simulink model "BaseEngineController_A02_bak2"  (Simulink 7.7)
settings: Solver=FixedStepDiscrete  StartTime=0.0  StopTime=10.0  RelTol=1e-3
PreLoadFcn:
  | try, eval([bdroot '_setup']); catch, eval('model_setup', ''); end
InitFcn:
  | try, eval([bdroot '_setup']); catch, eval('model_setup', ''); end
undefined symbols (not defined in the file or in its folder): ECU555, High, motohawk_build_version, motohawk_trunc_name, motohawk_vardec_config_group, motohawk_vardec_path, True
libraries: MotoHawk_lib x1081, AutoDoc_lib x609, motohawk_control_primitives_lib x132, motohawk_engine_lib x26, motohawk_annotations_lib x9, simulink x8, motohawk_control_primitives_motoshare_lib x1, simulink_extras x1
(part 22 of 22)

== system BaseEngineController_A02_bak2/Foreground/Control/Controller/Run/O2 Control/O2 PID Calibrations/O2 Control Switch Point Maps ==
  Sensors : Inport
  Bus Selector : BusSelector  OutputSignals=RawIndexes.MAP09Idx,RawIndexes.RPM09Idx
  Documentation_Calibrations_Probes_Block : AutoDoc_lib/Documentation_Calibrations_Probes_Block
  Documentation_Left_Border : AutoDoc_lib/Documentation_Left_Border  layout=Portrait
  Documentation_Right_Border : AutoDoc_lib/Documentation_Right_Border  layout=Portrait
  Documentation_Title_Block : AutoDoc_lib/Documentation_Title_Block
  motohawk_interpolation_2d : MotoHawk_lib/Lookup Tables/motohawk_interpolation_2d  nam='RichTargetDelta'  table_data=ones(9)  show_more=on  data_type=Inherit from 'Table Data'  storage=NonAdaptive  read_level=1  write_level=1  ovr_cals=on  use_help=on  help='Target on the rich side for perturbation.'  units='EquivRatio'  use_min_max=off  min_val=-inf  max_val=inf  use_precision=on  precision=''  gain=1  offset=0  exp=1  use_links=off  row_prelookup_block=''  col_prelookup_block=''  output_name=''  use_mototune_group=on  mototune_group=motohawk_vardec_path(gcb)
  motohawk_interpolation_2d1 : MotoHawk_lib/Lookup Tables/motohawk_interpolation_2d  nam='LeanTargetDelta'  table_data=ones(9)  show_more=on  data_type=Inherit from 'Table Data'  storage=NonAdaptive  read_level=1  write_level=1  ovr_cals=on  use_help=on  help='Target on the lean side for perturbation.'  units='EquivRatio'  use_min_max=off  min_val=-inf  max_val=inf  use_precision=on  precision=''  gain=1  offset=0  exp=1  use_links=off  row_prelookup_block=''  col_prelookup_block=''  output_name=''  use_mototune_group=on  mototune_group=motohawk_vardec_path(gcb)
  motohawk_interpolation_2d2 : MotoHawk_lib/Lookup Tables/motohawk_interpolation_2d  nam='RichSwPtDelta'  table_data=ones(9)  show_more=on  data_type=Inherit from 'Table Data'  storage=NonAdaptive  read_level=1  write_level=1  ovr_cals=on  use_help=on  help='If actual O2 reaches this point, switch the target to the LeanTargetDelta'  units='EquivRatio'  use_min_max=off  min_val=-inf  max_val=inf  use_precision=on  precision=''  gain=1  offset=0  exp=1  use_links=off  row_prelookup_block=''  col_prelookup_block=''  output_name=''  use_mototune_group=on  mototune_group=motohawk_vardec_path(gcb)
  motohawk_interpolation_2d3 : MotoHawk_lib/Lookup Tables/motohawk_interpolation_2d  nam='LeanSwPtDelta'  table_data=ones(9)  show_more=on  data_type=Inherit from 'Table Data'  storage=NonAdaptive  read_level=1  write_level=1  ovr_cals=on  use_help=on  help='If actual O2 reaches this point, switch the target to the RichTargetDelta'  units='EquivRatio'  use_min_max=off  min_val=-inf  max_val=inf  use_precision=on  precision=''  gain=1  offset=0  exp=1  use_links=off  row_prelookup_block=''  col_prelookup_block=''  output_name=''  use_mototune_group=on  mototune_group=motohawk_vardec_path(gcb)
  RichTargetDelta : Outport
  LeanTargetDelta : Outport  Port=2
  RichSwPtDelta : Outport  Port=3
  LeanSwPtDelta : Outport  Port=4
  wires:
  motohawk_interpolation_2d/1 -> RichTargetDelta/1  "RichEquivRatioTargetDelta"
  motohawk_interpolation_2d1/1 -> LeanTargetDelta/1  "LeanEquivRatioTargetDelta"
  motohawk_interpolation_2d2/1 -> RichSwPtDelta/1  "RichEquivRatioSwPtDelta"
  motohawk_interpolation_2d3/1 -> LeanSwPtDelta/1  "LeanEquivRatioSwPtDelta"
  Sensors/1 -> Bus Selector/1
  Bus Selector/1 -> motohawk_interpolation_2d3/1, motohawk_interpolation_2d2/1, motohawk_interpolation_2d1/1, motohawk_interpolation_2d/1  "<MAP09Idx>"
  Bus Selector/2 -> motohawk_interpolation_2d3/2, motohawk_interpolation_2d2/2, motohawk_interpolation_2d1/2, motohawk_interpolation_2d/2  "<RPM09Idx>"

== system BaseEngineController_A02_bak2/Foreground/Control/Controller/Run/O2 Control/O2 PID Controller/Closed-Loop O2 PID Controller ==
  O2Sensor : Inport
  TargetEquivRatio : Inport  Port=2
  ErrorGain : Inport  Port=3
  PGain : Inport  Port=4
  IGain : Inport  Port=5
  DGain : Inport  Port=6
  ITermUpperLimit : Inport  Port=7
  ITermLowerLimit : Inport  Port=8
  OutUpperLimit : Inport  Port=9
  OutLowerLimit : Inport  Port=10
  Reset : Inport  Port=11
  Constant : Constant  Value=0  OutDataTypeStr=Inherit: Inherit via back propagation
  Convert to Fuel Multiplier : Constant  OutDataTypeStr=Inherit: Inherit via back propagation
  Documentation_Calibrations_Probes_Block : AutoDoc_lib/Documentation_Calibrations_Probes_Block
  Documentation_Left_Border : AutoDoc_lib/Documentation_Left_Border  layout=Portrait
  Documentation_Right_Border : AutoDoc_lib/Documentation_Right_Border  layout=Portrait
  Documentation_Title_Block : AutoDoc_lib/Documentation_Title_Block
  Saturation : motohawk_control_primitives_lib/Saturation  SystemSampleTime=-1
  Sum : Sum  Inputs=++|  SaturateOnIntegerOverflow=on  InputSameDT=on
  Universal PID : motohawk_control_primitives_lib/Universal PID  Description=|  SystemSampleTime=-1  samp_time=.005
      | /* Name: ErrorGain Unit:mult ClassID:VAR StorageID:FLASH Access:RW4+RW3+NA2+NA1 TypeID:UINT4                   
      |    Gain:0.000000001 Min:0.000000000 Max:4.000000000 Format:10.9 UpdateID:REMOTE Help:"This gain is applied to "
      |    "the error term before it is used to calculate the P, I and D terms." VardecSig:6C */                       
      |                                                                                                                
      | /* Name: ITermLowerLim Unit:0 ClassID:VAR StorageID:FLASH Access:RW4+RW3+NA2+NA1 TypeID:SINT2 Gain:0.1         
      |    Min:-3276.8 Max:3276.7 Format:5.1 UpdateID:REMOTE Help:"Lower limit on the I-term value." VardecSig:6C */   
      |                                                                                                                
      | /* Name: ITermUpperLim ClassID:VAR StorageID:FLASH Access:RW4+RW3+NA2+NA1 TypeID:SINT2 Gain:0.1 Min:-3276.8    
      |    Max:3276.7 Format:5.1 UpdateID:REMOTE Help:"Upper limit on I-term value." VardecSig:6C */                   
      |                                                                                                                
      | /* Name: PIDOutLowerLim ClassID:VAR StorageID:FLASH Access:RW4+RW3+NA2+NA1 TypeID:SINT2 Gain:0.1               
      |    Min:-3276.8 Max:3276.7 Format:5.1 UpdateID:REMOTE Help:"Lower limit on PID output." VardecSig:6C */         
      |                                                                                                                
      | /* Name: PIDOutUpperLim ClassID:VAR StorageID:FLASH Access:RW4+RW3+NA2+NA1 TypeID:SINT2 Gain:0.1               
      |    Min:-3276.8 Max:3276.7 Format:3.1 UpdateID:REMOTE Help:"Upper limit on PID output." VardecSig:6C */
  motohawk_probe : MotoHawk_lib/Calibration & Probing Blocks/motohawk_probe  nam='LiquidO2Control_PTerm'  show_more=on  name_source=Use Parameter  read_level=1  view_as=Number  enum_struct={'Disabled', 'Enabled'}  vector_style=Wide Row  use_help_units=on  help='Closed-loop O2 control P-Term'  units='mult'  use_header_enum=off  row_header_enum={}  col_header_enum={}  use_precision=off  precision=''  gain=1  offset=0  exp=1  use_mototune_group=on  mototune_group=motohawk_vardec_path(gcb)
  motohawk_probe1 : MotoHawk_lib/Calibration & Probing Blocks/motohawk_probe  nam='LiquidO2Control_ITerm'  show_more=on  name_source=Use Parameter  read_level=1  view_as=Number  enum_struct={'Disabled', 'Enabled'}  vector_style=Wide Row  use_help_units=on  help='Closed-loop O2 control I-Term'  units='mult'  use_header_enum=off  row_header_enum={}  col_header_enum={}  use_precision=off  precision=''  gain=1  offset=0  exp=1  use_mototune_group=on  mototune_group=motohawk_vardec_path(gcb)
  motohawk_probe2 : MotoHawk_lib/Calibration & Probing Blocks/motohawk_probe  nam='LiquidO2Control_DTerm'  show_more=on  name_source=Use Parameter  read_level=1  view_as=Number  enum_struct={'Disabled', 'Enabled'}  vector_style=Wide Row  use_help_units=on  help='Closed-loop O2 control D-Term, for bank'  units='mult'  use_header_enum=off  row_header_enum={}  col_header_enum={}  use_precision=off  precision=''  gain=1  offset=0  exp=1  use_mototune_group=on  mototune_group=motohawk_vardec_path(gcb)
  motohawk_probe3 : MotoHawk_lib/Calibration & Probing Blocks/motohawk_probe  nam='LiquidO2Control_ErrorTerm'  show_more=on  name_source=Use Parameter  read_level=1  view_as=Number  enum_struct={'Disabled', 'Enabled'}  vector_style=Wide Row  use_help_units=on  help='Closed-loop O2 control error-term, for bank'  units='mult'  use_header_enum=off  row_header_enum={}  col_header_enum={}  use_precision=off  precision=''  gain=1  offset=0  exp=1  use_mototune_group=on  mototune_group=motohawk_vardec_path(gcb)
  Multiplier : Outport
  ITerm : Outport  Port=2
  wires:
  Reset/1 -> Universal PID/10
  Universal PID/3 -> motohawk_probe/1
  Universal PID/4 -> motohawk_probe1/1, ITerm/1
  Constant/1 -> Universal PID/7
  Universal PID/5 -> motohawk_probe2/1
  Saturation/1 -> Multiplier/1
  O2Sensor/1 -> Universal PID/1
  TargetEquivRatio/1 -> Universal PID/2
  ErrorGain/1 -> Universal PID/3
  PGain/1 -> Universal PID/4
  IGain/1 -> Universal PID/5
  DGain/1 -> Universal PID/6
  OutUpperLimit/1 -> Saturation/3
  Convert to Fuel Multiplier/1 -> Sum/1
  Universal PID/1 -> Sum/2
  Sum/1 -> Saturation/1
  ITermUpperLimit/1 -> Universal PID/9
  ITermLowerLimit/1 -> Universal PID/8
  OutLowerLimit/1 -> Saturation/2
  Universal PID/2 -> motohawk_probe3/1

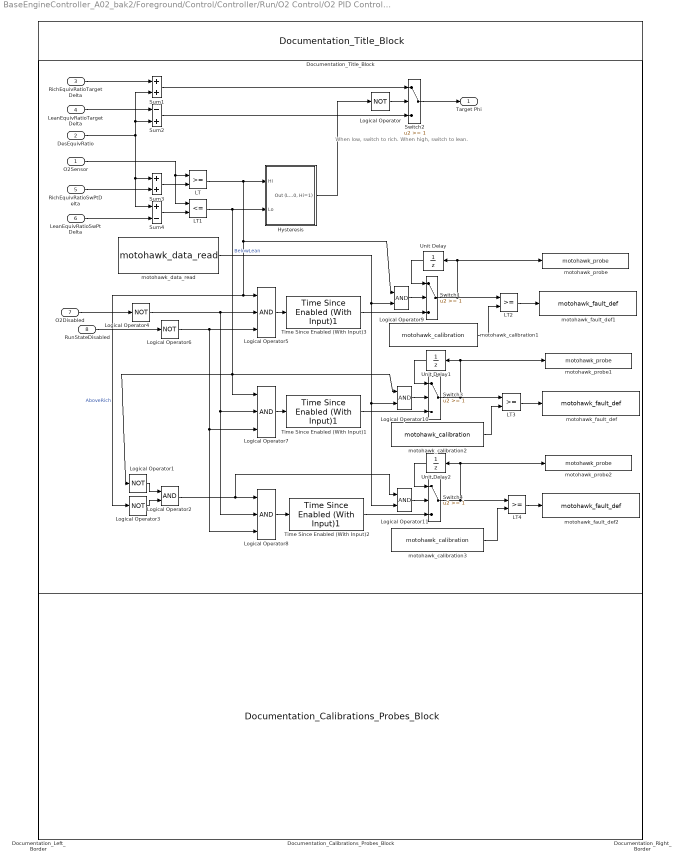
== system BaseEngineController_A02_bak2/Foreground/Control/Controller/Run/O2 Control/O2 PID Controller/Equivalence Ratio Perturbation ==
  note: When low, switch to rich.
      | When high, switch to lean.
  O2Sensor : Inport
  DesEquivRatio : Inport  Port=2
  RichEquivRatioTargetDelta : Inport  Port=3
  LeanEquivRatioTargetDelta : Inport  Port=4
  RichEquivRatioSwPtDelta : Inport  Port=5
  LeanEquivRatioSwPtDelta : Inport  Port=6
  O2Disabled : Inport  Port=7
  RunStateDisabled : Inport  Port=8
  Documentation_Calibrations_Probes_Block : AutoDoc_lib/Documentation_Calibrations_Probes_Block
  Documentation_Left_Border : AutoDoc_lib/Documentation_Left_Border  layout=Portrait
  Documentation_Right_Border : AutoDoc_lib/Documentation_Right_Border  layout=Portrait
  Documentation_Title_Block : AutoDoc_lib/Documentation_Title_Block
  Hysteresis : Subsystem
  LT{,2..4} (4 alike) : RelationalOperator  ZeroCross=off  InputSameDT=on
  LT1 : RelationalOperator  Operator=<=  ZeroCross=off  InputSameDT=on
  Logical Operator{,1,3..4,6} (5 alike) : Logic  Operator=NOT
  Logical Operator{2,9..11} (4 alike) : Logic
  Logical Operator5 : Logic  Inputs=3
  Logical Operator7 : Logic  Inputs=3
  Logical Operator8 : Logic  Inputs=3
  Sum1 : Sum  SaturateOnIntegerOverflow=on
  Sum2 : Sum  Inputs=-+  SaturateOnIntegerOverflow=on
  Sum3 : Sum  SaturateOnIntegerOverflow=on
  Sum4 : Sum  Inputs=+-  SaturateOnIntegerOverflow=on
  Switch{1..4} (4 alike) : Switch  Threshold=1  ZeroCross=off  InputSameDT=on
  Time Since Enabled (With Input)1 : motohawk_control_primitives_lib/Time Since Enabled (With Input)1  SystemSampleTime=-1  nam='O2LeanTimer'  samp_time=-1  init=0  upperlim=16000
  Time Since Enabled (With Input)2 : motohawk_control_primitives_lib/Time Since Enabled (With Input)1  SystemSampleTime=-1  nam='O2ActiveTimer'  samp_time=-1  init=0  upperlim=16000
  Time Since Enabled (With Input)3 : motohawk_control_primitives_lib/Time Since Enabled (With Input)1  SystemSampleTime=-1  nam='O2RichTimer'  samp_time=-1  init=0  upperlim=16000
  Unit Delay : UnitDelay  SampleTime=-1
  Unit Delay1 : UnitDelay  SampleTime=-1
  Unit Delay2 : UnitDelay  SampleTime=-1
  motohawk_calibration1 : MotoHawk_lib/Calibration & Probing Blocks/motohawk_calibration  nam='O2RichTimeThresh'  val=40  storage=Calibration  show_more=on  name_source=Use Parameter  data_type=Inherit from 'Default Value'  read_level=1  write_level=1  ovr_cals=on  view_as=Number  enum_struct={'Disabled', 'Enabled'}  vector_style=Wide Row  use_help_units=on  help='Time limit the O2 feedback can sit abovethe rich switch point before flagging O2FailedRich fault'  units='s'  use_header_enum=off  row_header_enum={}  col_header_enum={}  use_min_max=off  min_val=-inf  max_val=inf  use_precision=off  precision=''  gain=1  offset=0  exp=1  use_mototune_group=on  mototune_group=motohawk_vardec_path(gcb)
  motohawk_calibration2 : MotoHawk_lib/Calibration & Probing Blocks/motohawk_calibration  nam='O2LeanTimeThresh'  val=10  storage=Calibration  show_more=on  name_source=Use Parameter  data_type=Inherit from 'Default Value'  read_level=1  write_level=1  ovr_cals=on  view_as=Number  enum_struct={'Disabled', 'Enabled'}  vector_style=Wide Row  use_help_units=on  help='Time limit the O2 feedback can sit below the lean switch point before flagging O2FailedLean fault'  units='s'  use_header_enum=off  row_header_enum={}  col_header_enum={}  use_min_max=off  min_val=-inf  max_val=inf  use_precision=off  precision=''  gain=1  offset=0  exp=1  use_mototune_group=on  mototune_group=motohawk_vardec_path(gcb)
  motohawk_calibration3 : MotoHawk_lib/Calibration & Probing Blocks/motohawk_calibration  nam='O2ActiveTimeThresh'  val=40  storage=Calibration  show_more=on  name_source=Use Parameter  data_type=Inherit from 'Default Value'  read_level=1  write_level=1  ovr_cals=on  view_as=Number  enum_struct={'Disabled', 'Enabled'}  vector_style=Wide Row  use_help_units=on  help='Time limit O2 feedback can sit between rich and lean switch points before flagging O2NotActive fault'  units='s'  use_header_enum=off  row_header_enum={}  col_header_enum={}  use_min_max=off  min_val=-inf  max_val=inf  use_precision=off  precision=''  gain=1  offset=0  exp=1  use_mototune_group=on  mototune_group=motohawk_vardec_path(gcb)
  motohawk_data_read : MotoHawk_lib/Data Storage Blocks/motohawk_data_read  source=Lookup By Name  structure=Scalar  operation=Read scalar from location by index  sz_vec=4  sz_row=2  sz_col=3  typ=boolean  nam='DisableO2FaultAction'  checkNull=on  struct_inst_name='Struct1'  struct_nam='MyStruct'
  motohawk_fault_def : MotoHawk_lib/Fault Management Blocks/motohawk_fault_def  nam='O2FailedLean'  mode=Enabled  id=[]  x=25  y=50  input_suspected=on  indeterminate=off  increment_xy=on  downsample_count=1  ovr_cals=on  ovr_actions=on  show_actions=off  action1_name=''  action1_cond=(None)  action2_name=''  action2_cond=(None)  action3_name=''  action3_cond=(None)  action4_name=''  action4_cond=(None)
  motohawk_fault_def1 : MotoHawk_lib/Fault Management Blocks/motohawk_fault_def  nam='O2FailedRich'  mode=Enabled  id=[]  x=25  y=50  input_suspected=on  indeterminate=off  increment_xy=on  downsample_count=1  ovr_cals=on  ovr_actions=on  show_actions=off  action1_name=''  action1_cond=(None)  action2_name=''  action2_cond=(None)  action3_name=''  action3_cond=(None)  action4_name=''  action4_cond=(None)
  motohawk_fault_def2 : MotoHawk_lib/Fault Management Blocks/motohawk_fault_def  nam='O2NotActive'  mode=Enabled  id=[]  x=25  y=50  input_suspected=on  indeterminate=off  increment_xy=on  downsample_count=1  ovr_cals=on  ovr_actions=on  show_actions=off  action1_name=''  action1_cond=(None)  action2_name=''  action2_cond=(None)  action3_name=''  action3_cond=(None)  action4_name=''  action4_cond=(None)
  motohawk_probe : MotoHawk_lib/Calibration & Probing Blocks/motohawk_probe  nam='O2RichTime'  show_more=on  name_source=Use Parameter  read_level=1  view_as=Number  enum_struct={'Disabled', 'Enabled'}  vector_style=Wide Row  use_help_units=off  help=''  units=''  use_header_enum=off  row_header_enum={}  col_header_enum={}  use_precision=off  precision=''  gain=1  offset=0  exp=1  use_mototune_group=on  mototune_group=motohawk_vardec_path(gcb)
  motohawk_probe1 : MotoHawk_lib/Calibration & Probing Blocks/motohawk_probe  nam='O2LeanTime'  show_more=on  name_source=Use Parameter  read_level=1  view_as=Number  enum_struct={'Disabled', 'Enabled'}  vector_style=Wide Row  use_help_units=off  help=''  units=''  use_header_enum=off  row_header_enum={}  col_header_enum={}  use_precision=off  precision=''  gain=1  offset=0  exp=1  use_mototune_group=on  mototune_group=motohawk_vardec_path(gcb)
  motohawk_probe2 : MotoHawk_lib/Calibration & Probing Blocks/motohawk_probe  nam='O2NotActiveTime'  show_more=on  name_source=Use Parameter  read_level=1  view_as=Number  enum_struct={'Disabled', 'Enabled'}  vector_style=Wide Row  use_help_units=off  help=''  units=''  use_header_enum=off  row_header_enum={}  col_header_enum={}  use_precision=off  precision=''  gain=1  offset=0  exp=1  use_mototune_group=on  mototune_group=motohawk_vardec_path(gcb)
  Target Phi : Outport
  wires:
  Sum3/1 -> LT/2
  Sum4/1 -> LT1/2
  Switch2/1 -> Target Phi/1
  DesEquivRatio/1 -> Sum1/2, Sum2/2, Sum4/1, Sum3/1
  RichEquivRatioSwPtDelta/1 -> Sum3/2
  LeanEquivRatioSwPtDelta/1 -> Sum4/2
  Sum2/1 -> Switch2/3
  Sum1/1 -> Switch2/1
  LeanEquivRatioTargetDelta/1 -> Sum2/1
  RichEquivRatioTargetDelta/1 -> Sum1/1
  LT/1 -> Hysteresis/1(Hi), Logical Operator5/1, Logical Operator3/1, Logical Operator9/1  "AboveRich"
  LT1/1 -> Hysteresis/2(Lo), Logical Operator1/1, Logical Operator7/1, Logical Operator10/1  "BelowLean"
  Hysteresis/1("Out (Lo=0, Hi=1)") -> Logical Operator/1
  O2Sensor/1 -> LT1/1, LT/1
  Logical Operator/1 -> Switch2/2
  motohawk_calibration1/1 -> LT2/2
  LT2/1 -> motohawk_fault_def1/1
  LT3/1 -> motohawk_fault_def/1
  motohawk_calibration2/1 -> LT3/2
  LT4/1 -> motohawk_fault_def2/1
  motohawk_calibration3/1 -> LT4/2
  Logical Operator1/1 -> Logical Operator2/1
  Logical Operator3/1 -> Logical Operator2/2
  Logical Operator4/1 -> Logical Operator5/2, Logical Operator7/2, Logical Operator8/2
  O2Disabled/1 -> Logical Operator4/1
  RunStateDisabled/1 -> Logical Operator6/1
  Logical Operator6/1 -> Logical Operator5/3, Logical Operator7/3, Logical Operator8/3
  Logical Operator2/1 -> Logical Operator8/1, Logical Operator11/1
  Logical Operator5/1 -> Time Since Enabled (With Input)3/1
  Logical Operator7/1 -> Time Since Enabled (With Input)1/1
  Logical Operator8/1 -> Time Since Enabled (With Input)2/1
  Unit Delay/1 -> Switch1/1
  Time Since Enabled (With Input)3/1 -> Switch1/3
  Switch1/1 -> LT2/1, Unit Delay/1, motohawk_probe/1
  Logical Operator9/1 -> Switch1/2
  motohawk_data_read/1 -> Logical Operator9/2, Logical Operator10/2, Logical Operator11/2
  Unit Delay1/1 -> Switch3/1
  Switch3/1 -> LT3/1, Unit Delay1/1, motohawk_probe1/1
  Logical Operator10/1 -> Switch3/2
  Time Since Enabled (With Input)1/1 -> Switch3/3
  Unit Delay2/1 -> Switch4/1
  Switch4/1 -> LT4/1, Unit Delay2/1, motohawk_probe2/1
  Logical Operator11/1 -> Switch4/2
  Time Since Enabled (With Input)2/1 -> Switch4/3

== system BaseEngineController_A02_bak2/Foreground/Control/Controller/Stall/FAN Control/Hysterisis/Hysteresis ==
  note: Hi & !Lo => Hi
      | Lo & !Hi => Lo
      | Otherwise, Hold
  Hi : Inport
  Lo : Inport  Port=2
  Combinatorial  Logic : CombinatorialLogic  TruthTable=[0 0;0 1;1 1;0 0]
  Demux : Demux  Outputs=2
  Mux : Mux  Inputs=2
  Switch1 : Switch  Threshold=0.5  SaturateOnIntegerOverflow=on  InputSameDT=on
  Unit Delay : UnitDelay  SampleTime=-1
  "Out (Lo=0, Hi=1)" : Outport
  wires:
  Mux/1 -> Combinatorial  Logic/1
  Combinatorial  Logic/1 -> Demux/1
  Demux/1 -> Switch1/1
  Demux/2 -> Switch1/2
  Unit Delay/1 -> Switch1/3
  Switch1/1 -> Unit Delay/1, "Out (Lo=0, Hi=1)"/1
  Hi/1 -> Mux/1
  Lo/1 -> Mux/2

== system BaseEngineController_A02_bak2/Foreground/Control/Controller/Stall/FAN Control/Hysterisis1/Hysteresis ==
  note: Hi & !Lo => Hi
      | Lo & !Hi => Lo
      | Otherwise, Hold
  Hi : Inport
  Lo : Inport  Port=2
  Combinatorial  Logic : CombinatorialLogic  TruthTable=[0 0;0 1;1 1;0 0]
  Demux : Demux  Outputs=2
  Mux : Mux  Inputs=2
  Switch1 : Switch  Threshold=0.5  SaturateOnIntegerOverflow=on  InputSameDT=on
  Unit Delay : UnitDelay  SampleTime=-1
  "Out (Lo=0, Hi=1)" : Outport
  wires:
  Mux/1 -> Combinatorial  Logic/1
  Combinatorial  Logic/1 -> Demux/1
  Demux/1 -> Switch1/1
  Demux/2 -> Switch1/2
  Unit Delay/1 -> Switch1/3
  Switch1/1 -> Unit Delay/1, "Out (Lo=0, Hi=1)"/1
  Hi/1 -> Mux/1
  Lo/1 -> Mux/2

== system BaseEngineController_A02_bak2/Foreground/Control/Controller/Stall/FAN Control/Hysterisis2/Hysteresis ==
  note: Hi & !Lo => Hi
      | Lo & !Hi => Lo
      | Otherwise, Hold
  Hi : Inport
  Lo : Inport  Port=2
  Combinatorial  Logic : CombinatorialLogic  TruthTable=[0 0;0 1;1 1;0 0]
  Demux : Demux  Outputs=2
  Mux : Mux  Inputs=2
  Switch1 : Switch  Threshold=0.5  SaturateOnIntegerOverflow=on  InputSameDT=on
  Unit Delay : UnitDelay  SampleTime=-1
  "Out (Lo=0, Hi=1)" : Outport
  wires:
  Mux/1 -> Combinatorial  Logic/1
  Combinatorial  Logic/1 -> Demux/1
  Demux/1 -> Switch1/1
  Demux/2 -> Switch1/2
  Unit Delay/1 -> Switch1/3
  Switch1/1 -> Unit Delay/1, "Out (Lo=0, Hi=1)"/1
  Hi/1 -> Mux/1
  Lo/1 -> Mux/2

== system BaseEngineController_A02_bak2/Foreground/Control/Controller/Run/Fuel System Manager/Fuel Calculation/Calculate Fuel Flow/Calculate A/F Ratio ==
  Ethanol_xx [%] : Inport
  Documentation_Calibrations_Probes_Block : AutoDoc_lib/Documentation_Calibrations_Probes_Block
  Documentation_Left_Border : AutoDoc_lib/Documentation_Left_Border  layout=Portrait
  Documentation_Right_Border : AutoDoc_lib/Documentation_Right_Border  layout=Portrait
  Documentation_Title_Block : AutoDoc_lib/Documentation_Title_Block
  motohawk_table_1d : MotoHawk_lib/Lookup Tables/motohawk_table_1d  SystemSampleTime=-1  nam='DesAFRatio'  bp_nam=''  breakpoint_data=[0:10:100]  table_data=[14 14 14 14 14 14 14 14 14 14 14]  show_more=on  bp_data_type=Inherit from 'Breakpoint Data'  bp_storage=Constant  table_data_type=Inherit from 'Table Data'  storage=NonAdaptive  read_level=1  write_level=1  ovr_cals=on  use_help=on  help=''  bp_units='Ethanol%'  table_units='AFRatio'  use_min_max=on  bp_min_val=0  bp_max_val=100  min_val=0  max_val=20  use_precision=off  use_breakpoint=off  b_precision=''  b_gain=1  b_offset=0  b_exp=1  use_table=off  t_precision=''  t_gain=1  t_offset=0  t_exp=1  use_links=off  input_name=''  output_name=''  use_mototune_group=off  mototune_group=motohawk_vardec_path(gcb)
  Desired A/F Ratio : Outport
  wires:
  Ethanol_xx [%]/1 -> motohawk_table_1d/1
  motohawk_table_1d/1 -> Desired A/F Ratio/1

== system BaseEngineController_A02_bak2/Foreground/Control/Controller/Run/Fuel System Manager/Injector Manager/Delta TPS Transient Fueling/ECT Transient Fueling ==
  ECTIdx : Inport
  Enable : EnablePort
  Documentation_Calibrations_Probes_Block : AutoDoc_lib/Documentation_Calibrations_Probes_Block
  Documentation_Left_Border : AutoDoc_lib/Documentation_Left_Border  layout=Portrait
  Documentation_Right_Border : AutoDoc_lib/Documentation_Right_Border  layout=Portrait
  Documentation_Title_Block : AutoDoc_lib/Documentation_Title_Block
  motohawk_interpolation_1d : MotoHawk_lib/Lookup Tables/motohawk_interpolation_1d  nam='ECTTransFuelMult'  table_data=[1 1 1 1 1]  show_more=on  data_type=Inherit from 'Table Data'  storage=NonAdaptive  read_level=1  write_level=1  ovr_cals=on  use_help=on  help="'Transient fuel multiplier based on ECT.  Only engaged during transient fueling'"  units=''  use_min_max=off  min_val=-inf  max_val=inf  use_precision=off  precision=''  gain=1  offset=0  exp=1  use_links=off  prelookup_block=''  output_name=''  use_mototune_group=on  mototune_group=motohawk_vardec_path(gcb)
  Mult : Outport  OutputWhenDisabled=reset  InitialOutput=1
  wires:
  motohawk_interpolation_1d/1 -> Mult/1
  ECTIdx/1 -> motohawk_interpolation_1d/1

== system BaseEngineController_A02_bak2/Foreground/Control/Controller/Run/O2 Control/O2 PID Controller/Equivalence Ratio Perturbation/Hysteresis ==
  note: Hi & !Lo => Hi
      | Lo & !Hi => Lo
      | Otherwise, Hold
  Hi : Inport
  Lo : Inport  Port=2
  Combinatorial  Logic : CombinatorialLogic  TruthTable=[0 0;0 1;1 1;0 0]
  Demux : Demux  Outputs=2
  Documentation_Calibrations_Probes_Block : AutoDoc_lib/Documentation_Calibrations_Probes_Block
  Documentation_Left_Border : AutoDoc_lib/Documentation_Left_Border  layout=Portrait
  Documentation_Right_Border : AutoDoc_lib/Documentation_Right_Border  layout=Portrait
  Documentation_Title_Block : AutoDoc_lib/Documentation_Title_Block
  Mux : Mux  Inputs=2
  Switch1 : Switch  Threshold=0.5  SaturateOnIntegerOverflow=on  InputSameDT=on
  Unit Delay : UnitDelay  SampleTime=-1
  "Out (Lo=0, Hi=1)" : Outport
  wires:
  Mux/1 -> Combinatorial  Logic/1
  Combinatorial  Logic/1 -> Demux/1
  Demux/1 -> Switch1/1
  Demux/2 -> Switch1/2
  Unit Delay/1 -> Switch1/3
  Switch1/1 -> Unit Delay/1, "Out (Lo=0, Hi=1)"/1
  Hi/1 -> Mux/1
  Lo/1 -> Mux/2

== stateflow chart Foreground/Control/Controller/Run/DFCO/DesEquivRatioMod/Chart ==
  state default
    during: thresh = RampTime/5;
    state Normal
      during: EquivOut = EquivIn;
      SparkOut = 0;
    state DFCO_On
      during: EquivOut = 0;
      SparkOut = 0;
    state DFCO_Off
      entry: count = 0;
      EquivStart = EquivIn;
      during: count = count + 1;
      EquivOut = (EquivStart/thresh)*count;
      SparkOut = (SparkRet/thresh)*count;
    Normal -> DFCO_On : [DFCOState == 1]
    DFCO_Off -> Normal [order 1] : [count >= thresh]
    DFCO_On -> DFCO_Off : [DFCOState == 0]
    DFCO_Off -> DFCO_On [order 2] : [DFCOState == 1]
    (default) -> Normal
  (default) -> default
  data: DFCOState input double; EquivIn input double; EquivOut output double; SparkOut output double; SparkRet input double; RampTime input double; thresh local double; count local double; EquivStart local double

== stateflow chart Foreground/Control/VirtualSensors/RawProcessed/EngineState/Engine State Machine ==
  settings: executeAtInitialization=1
  state Stall/
    en:State=1;
  state Run/
    en:State=3;
  state Crank/
    en:State=2;
  (default) -> Stall
  Stall -> Crank : [Crank]
  Crank -> Run [order 2] : [Run]
  Run -> Stall : [Stall]
  Crank -> Stall [order 1] : [Stall]
  data: Stall input boolean; Crank input boolean; Run input boolean; State output uint8

== matlab function Foreground/Control/Controller/Run/MaxGovernor/MaxGovState/MaxGOV Stateflow ==
  data: OffToOn input double; ETCMode output double; OnToTrans input double; TransToOn input double; TransToOff input double; State input double; Reset output double; Hold output double; StateO output double
function [ETCMode,Reset,Hold,StateO] = fcn(OffToOn,OnToTrans,TransToOn,TransToOff,State)

if (State == 0 && OffToOn == 1)
    StateO = 1;
elseif (State == 1 && OnToTrans == 1)
    StateO=2;
elseif (State == 2 && TransToOn == 1)
    StateO=1;
elseif (State == 2 && TransToOff == 1)
    StateO=0;
else
    StateO=State;
end

if State == 0
    ETCMode=0;
    Hold=0;
    Reset=0;
elseif State == 1;
    ETCMode=1;
    Hold=0;
    Reset=0;
elseif State == 2;
    ETCMode=0;
    Hold=1;
    Reset=0;
else
    ETCMode=0;
    Hold=0;
    Reset=0;
end

== stateflow chart Main Power Relay/Main Power Relay/ECUP Latch ==
  settings: executeAtInitialization=1
  state PowerDown/
    en:MPRDState=0;
  state PowerUp/
    en:MPRDState=1;
  (default) -> PowerDown
  PowerUp -> PowerDown : [!ECUP&&ShutDownTasks]
  PowerDown -> PowerUp : [ECUP]
  data: ECUP input boolean; ShutDownTasks input boolean; MPRDState output boolean

== stateflow chart Foreground/Control/ActuatorCharacterization/Electronic Throttle Controller/ETC Test  Manager ==
  settings: executeAtInitialization=1
  state PassThrough/
    en:DC_Override=0;
    en:TestComplete=0;
    en:SetpointMode=0;
    en:TestTime=0;
  state LowAdapt/
    en:DC_Override=0;
    en:TestComplete=0;
    en:SetpointMode=1;
    en:TestTime=0;
  state SpringTestSetpoint/
    en:DC_Override=0;
    en:TestComplete=0;
    en:SetpointMode=2;
    en:TestTime=0;
  state SpringTest/
    en:DC_Override=1;
    en:TestComplete=0;
    en:SetpointMode=0;
    en:TestTime=0;
    en:TimerOld=Timer;
  state SpringTestComplete/
    en:DC_Override=1;
    en:TestComplete=1;
    en:SetpointMode=0;
    en:TestTime=Timer-TimerOld;
  state ForcedShutdown/
    en:DC_Override=1;
    en:TestComplete=1;
    en:SetpointMode=0;
    en:TestTime=0;
  (default) -> PassThrough
  PassThrough -> LowAdapt : [ECUP_Off]
  LowAdapt -> SpringTestSetpoint [order 2] : [ECUP_Off&&LowAdaptError]
  SpringTestSetpoint -> SpringTest [order 2] : [ECUP_Off&&TPSHiThresh]
  SpringTest -> SpringTestComplete [order 2] : [ECUP_Off&&TPSLoThresh]
  LowAdapt -> PassThrough [order 3] : [!ECUP_Off]
  SpringTestSetpoint -> PassThrough [order 1] : [!ECUP_Off]
  SpringTestComplete -> PassThrough : [!ECUP_Off]
  SpringTest -> PassThrough [order 3] : [!ECUP_Off]
  SpringTest -> ForcedShutdown [order 1] : [Timer>5]
  LowAdapt -> ForcedShutdown [order 1] : [Timer>5]
  SpringTestSetpoint -> ForcedShutdown [order 3] : [Timer>5]
  ForcedShutdown -> SpringTestComplete : [!ECUP_Off]
  data: TestTime output double; ECUP_Off input boolean; DC_Override output int8; Timer input double; LowAdaptError input boolean; TPSHiThresh input boolean; TPSLoThresh input boolean; TimerOld local double; TestComplete output boolean; SetpointMode output int8

== matlab function Foreground/Outputs/Coil Control/Coil Enable Manager/EST Enable TDC Counter/EST Enable TDC Counter Stateflow ==
  data: CounterEnabled input double; CounterReset output double; EngineState input double; State input double; ESTEnable output double; StateO output double
function [CounterReset,ESTEnable,StateO] = fcn(CounterEnabled,EngineState,State)

if (State == 0 && CounterEnabled == 1)
    StateO=1;
elseif (State == 1 && EngineState == 2 && CounterEnabled == 1)
    StateO=2;
elseif (State == 2 && EngineState ==3)
    StateO=3;
elseif (State == 2 && EngineState == 1)
    StateO=0;
elseif (State ==3 && EngineState == 1)
    StateO=0;
else
    StateO=State;
end

if State == 0
   ESTEnable=0;
   CounterReset=0;
elseif State == 1
    ESTEnable=1;
    CounterReset=0;
elseif State == 2
    ESTEnable=1;
    CounterReset=1;
elseif State == 3
    ESTEnable=1;
    CounterReset=1;
else
    ESTEnable=0;
    CounterReset=0;
end

== stateflow chart Foreground/Control/Controller/Run/MinGovernor/Idle State Determination/IdleStateMachine ==
  state OffIdle/
    en:IdleState = 3;
  state Flaring/
    en:IdleState = 1;
  state OnIdle/
    en:IdleState = 2;
  (default) -> Flaring
  Flaring -> OffIdle [order 1] : [ThrCmdHigh || !IdleEnable]
  OffIdle -> OnIdle : [IdleEnable && (RPMLow || (RPMInRange && ...
      | ThrCmdLow)) || APPTimeThresh]
  Flaring -> OnIdle [order 2] : [RPMFalling && ThrCmdLow]
  OnIdle -> OffIdle : [!RPMLow&&(ThrCmdHigh || !IdleEnable)]
  data: IdleEnable input boolean; RPMFalling input boolean; RPMInRange input boolean; RPMLow input boolean; ThrCmdLow input boolean; ThrCmdHigh input boolean; IdleState output uint8; APPTimeThresh input boolean

== stateflow chart Foreground/Control/VirtualSensors/AirCalcs/Baro Stall State Delay ==
  settings: executeAtInitialization=1
  state Stall/
    en:Enable=1;
  state CrankRun/
    en:Enable=0;
  state StallDelay/
    en:Enable=0;
    en:Timer=0;
    en:TOld=0;
    during:Timer=TOld+dT;
    during:TOld=Timer;
  (default) -> Stall
  Stall -> CrankRun : [EngineState>1]
  CrankRun -> StallDelay : [EngineState==1]
  StallDelay -> Stall [order 2] : [EngineState==1&Timer>TimeThresh]
  StallDelay -> CrankRun [order 1] : [EngineState>1]
  data: EngineState input uint8; TimeThresh input double; dT input double; Timer output double; Enable output boolean; TOld local double
